simulink model "MX_Gateway"  (Simulink 9.0)
settings: Solver=FixedStepDiscrete  StartTime=0.0  StopTime=100  FixedStep=0.01  RelTol=1e-3
values from neighbouring files (symbols this model uses):
  ACTIVE = Bobcat.Parameter; CreateDefine( ACTIVE                         ,1                          ,'Define' ,'defines.h' )    [defines.m]
  ACTIVE_MODE_ONE = Bobcat.Parameter; CreateDefine( ACTIVE_MODE_ONE                ,19                         ,'Define' ,'defines.h' )    [defines.m]
  AUTHENTICATED = Bobcat.Parameter; CreateDefine( AUTHENTICATED                  ,1                          ,'Define' ,'defines.h' )    [defines.m]
  AUX_EMEA_CONTROLS = Bobcat.Parameter; CreateDefine( AUX_EMEA_CONTROLS  ,1   ,'Define' ,'defines.h' )    [enums.m]
  AUX_FLOW_HIGH = Bobcat.Parameter; CreateDefine( AUX_FLOW_HIGH           ,4  ,'Define' ,'defines.h' )    [enums.m]
  AUX_FLOW_INACTIVE = Bobcat.Parameter; CreateDefine( AUX_FLOW_INACTIVE       ,0  ,'Define' ,'defines.h' )    [enums.m]
  AUX_FLOW_LOW = Bobcat.Parameter; CreateDefine( AUX_FLOW_LOW            ,1  ,'Define' ,'defines.h' )    [enums.m]
  AUX_FLOW_MEDIUM = Bobcat.Parameter; CreateDefine( AUX_FLOW_MEDIUM         ,2  ,'Define' ,'defines.h' )    [enums.m]
  AUX_FLOW_STANDARD = Bobcat.Parameter; CreateDefine( AUX_FLOW_STANDARD       ,3  ,'Define' ,'defines.h' )    [enums.m]
  AUX_NON_FUNCTIONAL = Bobcat.Parameter; CreateDefine( AUX_NON_FUNCTIONAL ,255 ,'Define' ,'defines.h' )    [enums.m]
  BASE_ACTIVE = Bobcat.Parameter; CreateDefine( BASE_ACTIVE                    ,253                        ,'Define' ,'defines.h' )    [defines.m]
  BASE_DETENT = Bobcat.Parameter; CreateDefine( BASE_DETENT                    ,1                          ,'Define' ,'defines.h' )    [defines.m]
  BEEP_NONE = Bobcat.Parameter; CreateDefine( BEEP_NONE                      ,255                        ,'Define' ,'defines.h' )    [defines.m]
  CD_AIR_FILTER_DEBOUNCE_NORMAL = Bobcat.Parameter; CreateAliasCD( CD_AIR_FILTER_DEBOUNCE_NORMAL                        ,uint16(500)                ,'ConstantDataPointer' ,'CONSTANT_DATA' ,'AIR_FILTER_DEBOUNCE_NORMAL')    [cdata.m]    (also in hc12_cdata.m; m4_cdata.m)
  CD_AIR_FILTER_DEBOUNCE_NOT_CONNECTED = Bobcat.Parameter; CreateAliasCD( CD_AIR_FILTER_DEBOUNCE_NOT_CONNECTED                 ,uint16(3000)               ,'ConstantDataPointer' ,'CONSTANT_DATA' ,'AIR_FILTER_DEBOUNCE_NOT_CONNECTED')    [cdata.m]    (also in hc12_cdata.m; m4_cdata.m)
  CD_AIR_FILTER_DEBOUNCE_PLUGGED = Bobcat.Parameter; CreateAliasCD( CD_AIR_FILTER_DEBOUNCE_PLUGGED                       ,uint16(3000)               ,'ConstantDataPointer' ,'CONSTANT_DATA' ,'AIR_FILTER_DEBOUNCE_PLUGGED')    [cdata.m]    (also in hc12_cdata.m; m4_cdata.m)
  CD_AIR_FILTER_FUNCTION = Bobcat.Parameter; CreateAliasCD( CD_AIR_FILTER_FUNCTION                               ,uint8(255)                 ,'ConstantDataPointer' ,'CONSTANT_DATA' ,'AIR_FILTER_FUNCTION')    [cdata.m]    (also in hc12_cdata.m; m4_cdata.m)
  CD_AIR_FILTER_PLUGGED_WEIGHT = Bobcat.Parameter; CreateAliasCD( CD_AIR_FILTER_PLUGGED_WEIGHT                         ,uint8(2)                   ,'ConstantDataPointer' ,'CONSTANT_DATA' ,'AIR_FILTER_PLUGGED_WEIGHT')    [cdata.m]    (also in hc12_cdata.m; m4_cdata.m)
  CD_AIR_FILTER_UNPLUGGED_WEIGHT = Bobcat.Parameter; CreateAliasCD( CD_AIR_FILTER_UNPLUGGED_WEIGHT                       ,uint8(1)                   ,'ConstantDataPointer' ,'CONSTANT_DATA' ,'AIR_FILTER_UNPLUGGED_WEIGHT')    [cdata.m]    (also in hc12_cdata.m; m4_cdata.m)
  CD_AUTOIDLE_ARMBAR_DELAY = Bobcat.Parameter; CreateAliasCD( CD_AUTOIDLE_ARMBAR_DELAY                             ,uint8(2)                   ,'ConstantDataPointer' ,'CONSTANT_DATA' ,'AUTOIDLE_ARMBAR_DELAY')    [cdata.m]    (also in hc12_cdata.m; m4_cdata.m)
  CD_AUTOIDLE_FUNCTION = Bobcat.Parameter; CreateAliasCD( CD_AUTOIDLE_FUNCTION                                 ,uint8(255)                 ,'ConstantDataPointer' ,'CONSTANT_DATA' ,'AUTOIDLE_FUNCTION')    [cdata.m]    (also in hc12_cdata.m; m4_cdata.m)
  CD_AUTOIDLE_IDLE_DELAY = Bobcat.Parameter; CreateAliasCD( CD_AUTOIDLE_IDLE_DELAY                               ,uint8(40)                  ,'ConstantDataPointer' ,'CONSTANT_DATA' ,'AUTOIDLE_IDLE_DELAY')    [cdata.m]    (also in hc12_cdata.m; m4_cdata.m)
  CD_AUTOIDLE_LOW_IDLE_START = Bobcat.Parameter; CreateAliasCD( CD_AUTOIDLE_LOW_IDLE_START                           ,uint8(255)                 ,'ConstantDataPointer' ,'CONSTANT_DATA' ,'AUTOIDLE_LOW_IDLE_START')    [cdata.m]    (also in hc12_cdata.m; m4_cdata.m)
  CD_AUTOIDLE_PRESSURE_OFF = Bobcat.Parameter; CreateAliasCD( CD_AUTOIDLE_PRESSURE_OFF                             ,uint16(300)                ,'ConstantDataPointer' ,'CONSTANT_DATA' ,'AUTOIDLE_PRESSURE_OFF')    [cdata.m]    (also in hc12_cdata.m; m4_cdata.m)
  CD_AUTOIDLE_PRESSURE_ON = Bobcat.Parameter; CreateAliasCD( CD_AUTOIDLE_PRESSURE_ON                              ,uint16(270)                ,'ConstantDataPointer' ,'CONSTANT_DATA' ,'AUTOIDLE_PRESSURE_ON')    [cdata.m]    (also in hc12_cdata.m; m4_cdata.m)
  CD_AUTOSHIFT_FUNCTION = Bobcat.Parameter; CreateAliasCD( CD_AUTOSHIFT_FUNCTION                                ,uint8(255)                 ,'ConstantDataPointer' ,'CONSTANT_DATA' ,'AUTOSHIFT_FUNCTION')    [cdata.m]    (also in hc12_cdata.m; m4_cdata.m)
  CD_AUTOSHIFT_PHIGH1 = Bobcat.Parameter; CreateAliasCD( CD_AUTOSHIFT_PHIGH1                                  ,uint16(205)                ,'ConstantDataPointer' ,'CONSTANT_DATA' ,'AUTOSHIFT_PHIGH1')    [cdata.m]    (also in hc12_cdata.m; m4_cdata.m)
  CD_AUTOSHIFT_PHIGH2 = Bobcat.Parameter; CreateAliasCD( CD_AUTOSHIFT_PHIGH2                                  ,uint16(193)                ,'ConstantDataPointer' ,'CONSTANT_DATA' ,'AUTOSHIFT_PHIGH2')    [cdata.m]    (also in hc12_cdata.m; m4_cdata.m)
  CD_AUTOSHIFT_PHIGH_TEMP1 = Bobcat.Parameter; CreateAliasCD( CD_AUTOSHIFT_PHIGH_TEMP1                             ,int16(70)                  ,'ConstantDataPointer' ,'CONSTANT_DATA' ,'AUTOSHIFT_PHIGH_TEMP1')    [cdata.m]    (also in hc12_cdata.m; m4_cdata.m)
  CD_AUTOSHIFT_PHIGH_TEMP2 = Bobcat.Parameter; CreateAliasCD( CD_AUTOSHIFT_PHIGH_TEMP2                             ,int16(160)                 ,'ConstantDataPointer' ,'CONSTANT_DATA' ,'AUTOSHIFT_PHIGH_TEMP2')    [cdata.m]    (also in hc12_cdata.m; m4_cdata.m)
  CD_AUTOSHIFT_PLOW1 = Bobcat.Parameter; CreateAliasCD( CD_AUTOSHIFT_PLOW1                                   ,uint16(130)                ,'ConstantDataPointer' ,'CONSTANT_DATA' ,'AUTOSHIFT_PLOW1')    [cdata.m]    (also in hc12_cdata.m; m4_cdata.m)
  CD_AUTOSHIFT_PLOW2 = Bobcat.Parameter; CreateAliasCD( CD_AUTOSHIFT_PLOW2                                   ,uint16(100)                ,'ConstantDataPointer' ,'CONSTANT_DATA' ,'AUTOSHIFT_PLOW2')    [cdata.m]    (also in hc12_cdata.m; m4_cdata.m)
  CD_AUTOSHIFT_PLOW_TEMP1 = Bobcat.Parameter; CreateAliasCD( CD_AUTOSHIFT_PLOW_TEMP1                              ,int16(20)                  ,'ConstantDataPointer' ,'CONSTANT_DATA' ,'AUTOSHIFT_PLOW_TEMP1')    [cdata.m]    (also in hc12_cdata.m; m4_cdata.m)
  CD_AUTOSHIFT_PLOW_TEMP2 = Bobcat.Parameter; CreateAliasCD( CD_AUTOSHIFT_PLOW_TEMP2                              ,int16(80)                  ,'ConstantDataPointer' ,'CONSTANT_DATA' ,'AUTOSHIFT_PLOW_TEMP2')    [cdata.m]    (also in hc12_cdata.m; m4_cdata.m)
  CD_AUTOSHIFT_TRAVEL_DELAY = Bobcat.Parameter; CreateAliasCD( CD_AUTOSHIFT_TRAVEL_DELAY                            ,uint8(32)                  ,'ConstantDataPointer' ,'CONSTANT_DATA' ,'AUTOSHIFT_TRAVEL_DELAY')    [cdata.m]    (also in hc12_cdata.m; m4_cdata.m)
  CD_AUX2_CRACKPOINT_CURRENT = Bobcat.Parameter; CreateAliasCD( CD_AUX2_CRACKPOINT_CURRENT                           ,fi(0,0,16,12,'hex','0c28') ,'ConstantDataPointer' ,'CONSTANT_DATA' ,'AUX2_CRACKPOINT_CURRENT')    [cdata.m]    (also in hc12_cdata.m; m4_cdata.m)
  CD_AUX2_FLOW_LOW_FUNCTION = Bobcat.Parameter; CreateAliasCD( CD_AUX2_FLOW_LOW_FUNCTION                            ,uint8(255)                 ,'ConstantDataPointer' ,'CONSTANT_DATA' ,'AUX2_FLOW_LOW_FUNCTION')    [cdata.m]    (also in hc12_cdata.m; m4_cdata.m)
  CD_AUX2_FLOW_MED_FUNCTION = Bobcat.Parameter; CreateAliasCD( CD_AUX2_FLOW_MED_FUNCTION                            ,uint8(255)                 ,'ConstantDataPointer' ,'CONSTANT_DATA' ,'AUX2_FLOW_MED_FUNCTION')    [cdata.m]    (also in hc12_cdata.m; m4_cdata.m)
  CD_AUX2_HI_FLOW_PERCENT = Bobcat.Parameter; CreateAliasCD( CD_AUX2_HI_FLOW_PERCENT                              ,fi(0,0,16,16,'hex','ffff') ,'ConstantDataPointer' ,'CONSTANT_DATA' ,'AUX2_HI_FLOW_PERCENT')    [cdata.m]    (also in hc12_cdata.m; m4_cdata.m)
  CD_AUX2_LOW_FLOW_PERCENT = Bobcat.Parameter; CreateAliasCD( CD_AUX2_LOW_FLOW_PERCENT                             ,fi(0,0,16,16,'hex','7333') ,'ConstantDataPointer' ,'CONSTANT_DATA' ,'AUX2_LOW_FLOW_PERCENT')    [cdata.m]    (also in hc12_cdata.m; m4_cdata.m)
  CD_AUX2_MAX_FLOW_CURRENT = Bobcat.Parameter; CreateAliasCD( CD_AUX2_MAX_FLOW_CURRENT                             ,fi(0,0,16,12,'hex','14cc') ,'ConstantDataPointer' ,'CONSTANT_DATA' ,'AUX2_MAX_FLOW_CURRENT')    [cdata.m]    (also in hc12_cdata.m; m4_cdata.m)
  CD_AUX2_MED_FLOW_PERCENT = Bobcat.Parameter; CreateAliasCD( CD_AUX2_MED_FLOW_PERCENT                             ,fi(0,0,16,16,'hex','9999') ,'ConstantDataPointer' ,'CONSTANT_DATA' ,'AUX2_MED_FLOW_PERCENT')    [cdata.m]    (also in hc12_cdata.m; m4_cdata.m)
  CD_AUX_CRACKPOINT_CURRENT = Bobcat.Parameter; CreateAliasCD( CD_AUX_CRACKPOINT_CURRENT                            ,fi(0,0,16,12,'hex','0000') ,'ConstantDataPointer' ,'CONSTANT_DATA' ,'AUX_CRACKPOINT_CURRENT')    [cdata.m]    (also in hc12_cdata.m; m4_cdata.m)
  CD_AUX_DETENT_FLASH_TIME = Bobcat.Parameter; CreateAliasCD( CD_AUX_DETENT_FLASH_TIME                             ,uint16(72)                 ,'ConstantDataPointer' ,'CONSTANT_DATA' ,'AUX_DETENT_FLASH_TIME')    [cdata.m]    (also in hc12_cdata.m; m4_cdata.m)
  CD_AUX_DETENT_HOLD_TIME = Bobcat.Parameter; CreateAliasCD( CD_AUX_DETENT_HOLD_TIME                              ,uint16(100)                ,'ConstantDataPointer' ,'CONSTANT_DATA' ,'AUX_DETENT_HOLD_TIME')    [cdata.m]    (also in hc12_cdata.m; m4_cdata.m)
  CD_AUX_FULL_ON_AUX1 = Bobcat.Parameter; CreateAliasCD( CD_AUX_FULL_ON_AUX1                                  ,fi(0,0,16,12,'hex','10cd') ,'ConstantDataPointer' ,'CONSTANT_DATA' ,'AUX_FULL_ON_AUX1')    [cdata.m]    (also in hc12_cdata.m; m4_cdata.m)
  CD_AUX_FULL_ON_AUX2 = Bobcat.Parameter; CreateAliasCD( CD_AUX_FULL_ON_AUX2                                  ,fi(0,0,16,12,'hex','139d') ,'ConstantDataPointer' ,'CONSTANT_DATA' ,'AUX_FULL_ON_AUX2')    [cdata.m]    (also in hc12_cdata.m; m4_cdata.m)
  CD_AUX_FULL_ON_AUX3 = Bobcat.Parameter; CreateAliasCD( CD_AUX_FULL_ON_AUX3                                  ,fi(0,0,16,12,'hex','17d7') ,'ConstantDataPointer' ,'CONSTANT_DATA' ,'AUX_FULL_ON_AUX3')    [cdata.m]    (also in hc12_cdata.m; m4_cdata.m)
  CD_AUX_FUNCTION = Bobcat.Parameter; CreateAliasCD( CD_AUX_FUNCTION                                      ,uint8(0)                   ,'ConstantDataPointer' ,'CONSTANT_DATA' ,'AUX_FUNCTION')    [cdata.m]    (also in hc12_cdata.m; m4_cdata.m)
  CD_AUX_RATE_LIMIT_CRACKPOINT = Bobcat.Parameter; CreateAliasCD( CD_AUX_RATE_LIMIT_CRACKPOINT                         ,fi(0,0,16,12,'hex','0800') ,'ConstantDataPointer' ,'CONSTANT_DATA' ,'AUX_RATE_LIMIT_CRACKPOINT')    [cdata.m]    (also in hc12_cdata.m; m4_cdata.m)
  CD_AUX_RATE_LIMIT_CRACKPOINT_RATE = Bobcat.Parameter; CreateAliasCD( CD_AUX_RATE_LIMIT_CRACKPOINT_RATE                    ,fi(0,1,32,12,'int',102400) ,'ConstantDataPointer' ,'CONSTANT_DATA' ,'AUX_RATE_LIMIT_CRACKPOINT_RATE')    [cdata.m]    (also in hc12_cdata.m; m4_cdata.m)
  CD_AUX_RATE_LIMIT_FUNCTION = Bobcat.Parameter; CreateAliasCD( CD_AUX_RATE_LIMIT_FUNCTION                           ,uint8(255)                 ,'ConstantDataPointer' ,'CONSTANT_DATA' ,'AUX_RATE_LIMIT_FUNCTION')    [cdata.m]    (also in hc12_cdata.m; m4_cdata.m)
  CD_AUX_RATE_LIMIT_MAX_ENG_SPD = Bobcat.Parameter; CreateAliasCD( CD_AUX_RATE_LIMIT_MAX_ENG_SPD                        ,uint16(1600)               ,'ConstantDataPointer' ,'CONSTANT_DATA' ,'AUX_RATE_LIMIT_MAX_ENG_SPD')    [cdata.m]    (also in hc12_cdata.m; m4_cdata.m)
  CD_AUX_RATE_LIMIT_MIN_ENG_SPD = Bobcat.Parameter; CreateAliasCD( CD_AUX_RATE_LIMIT_MIN_ENG_SPD                        ,uint16(100)                ,'ConstantDataPointer' ,'CONSTANT_DATA' ,'AUX_RATE_LIMIT_MIN_ENG_SPD')    [cdata.m]    (also in hc12_cdata.m; m4_cdata.m)
  CD_AUX_RATE_LIMIT_NORMAL_RATE = Bobcat.Parameter; CreateAliasCD( CD_AUX_RATE_LIMIT_NORMAL_RATE                        ,fi(0,1,32,12,'int',2048)   ,'ConstantDataPointer' ,'CONSTANT_DATA' ,'AUX_RATE_LIMIT_NORMAL_RATE')    [cdata.m]    (also in hc12_cdata.m; m4_cdata.m)
  CD_AUXPOT_CAL_DEADBAND_HIGH = Bobcat.Parameter; CreateAliasCD( CD_AUXPOT_CAL_DEADBAND_HIGH                          ,uint16(574)                ,'ConstantDataPointer' ,'CONSTANT_DATA' ,'AUXPOT_CAL_DEADBAND_HIGH')    [cdata.m]    (also in hc12_cdata.m; m4_cdata.m)
  CD_AUXPOT_CAL_DEADBAND_LOW = Bobcat.Parameter; CreateAliasCD( CD_AUXPOT_CAL_DEADBAND_LOW                           ,uint16(424)                ,'ConstantDataPointer' ,'CONSTANT_DATA' ,'AUXPOT_CAL_DEADBAND_LOW')    [cdata.m]    (also in hc12_cdata.m; m4_cdata.m)
  CD_AUXPOT_CENTER_RATIO = Bobcat.Parameter; CreateAliasCD( CD_AUXPOT_CENTER_RATIO                               ,uint16(500)                ,'ConstantDataPointer' ,'CONSTANT_DATA' ,'AUXPOT_CENTER_RATIO')    [cdata.m]    (also in hc12_cdata.m; m4_cdata.m)
  CD_AUXPOT_DEADBAND = Bobcat.Parameter; CreateAliasCD( CD_AUXPOT_DEADBAND                                   ,uint8(38)                  ,'ConstantDataPointer' ,'CONSTANT_DATA' ,'AUXPOT_DEADBAND')    [cdata.m]    (also in hc12_cdata.m; m4_cdata.m)
  CD_AUXPOT_FUNCTION = Bobcat.Parameter; CreateAliasCD( CD_AUXPOT_FUNCTION                                   ,uint8(0)                   ,'ConstantDataPointer' ,'CONSTANT_DATA' ,'AUXPOT_FUNCTION')    [cdata.m]    (also in hc12_cdata.m; m4_cdata.m)
  CD_AUXPOT_RATIO_GAIN = Bobcat.Parameter; CreateAliasCD( CD_AUXPOT_RATIO_GAIN                                 ,uint16(1000)               ,'ConstantDataPointer' ,'CONSTANT_DATA' ,'AUXPOT_RATIO_GAIN')    [cdata.m]    (also in hc12_cdata.m; m4_cdata.m)
  CD_CAN_THROTTLE_FUNCTION = Bobcat.Parameter; CreateAliasCD( CD_CAN_THROTTLE_FUNCTION                             ,uint8(255)                 ,'ConstantDataPointer' ,'CONSTANT_DATA' ,'CAN_THROTTLE_FUNCTION')    [cdata.m]    (also in hc12_cdata.m; m4_cdata.m)
  CD_CAN_THROTTLE_REVERSE = Bobcat.Parameter; CreateAliasCD( CD_CAN_THROTTLE_REVERSE                              ,uint8(255)                 ,'ConstantDataPointer' ,'CONSTANT_DATA' ,'CAN_THROTTLE_REVERSE')    [cdata.m]    (also in hc12_cdata.m; m4_cdata.m)
  CD_CONSOLE_DEBOUNCE_ABSENT = Bobcat.Parameter; CreateAliasCD( CD_CONSOLE_DEBOUNCE_ABSENT                           ,uint16(12)                 ,'ConstantDataPointer' ,'CONSTANT_DATA' ,'CONSOLE_DEBOUNCE_ABSENT')    [cdata.m]    (also in hc12_cdata.m; m4_cdata.m)
  CD_CONSOLE_DEBOUNCE_OORH = Bobcat.Parameter; CreateAliasCD( CD_CONSOLE_DEBOUNCE_OORH                             ,uint16(12)                 ,'ConstantDataPointer' ,'CONSTANT_DATA' ,'CONSOLE_DEBOUNCE_OORH')    [cdata.m]    (also in hc12_cdata.m; m4_cdata.m)
  CD_CONSOLE_DEBOUNCE_OORL = Bobcat.Parameter; CreateAliasCD( CD_CONSOLE_DEBOUNCE_OORL                             ,uint16(12)                 ,'ConstantDataPointer' ,'CONSTANT_DATA' ,'CONSOLE_DEBOUNCE_OORL')    [cdata.m]    (also in hc12_cdata.m; m4_cdata.m)
  CD_CONSOLE_DEBOUNCE_PRESENT = Bobcat.Parameter; CreateAliasCD( CD_CONSOLE_DEBOUNCE_PRESENT                          ,uint16(12)                 ,'ConstantDataPointer' ,'CONSTANT_DATA' ,'CONSOLE_DEBOUNCE_PRESENT')    [cdata.m]    (also in hc12_cdata.m; m4_cdata.m)
  CD_CONSOLE_FUNCTION = Bobcat.Parameter; CreateAliasCD( CD_CONSOLE_FUNCTION                                  ,uint8(0)                   ,'ConstantDataPointer' ,'CONSTANT_DATA' ,'CONSOLE_FUNCTION')    [cdata.m]    (also in hc12_cdata.m; m4_cdata.m)
  CD_CONSOLE_OORH = Bobcat.Parameter; CreateAliasCD( CD_CONSOLE_OORH                                      ,uint16(740)                ,'ConstantDataPointer' ,'CONSTANT_DATA' ,'CONSOLE_OORH')    [cdata.m]    (also in hc12_cdata.m; m4_cdata.m)
  CD_CONSOLE_OORL = Bobcat.Parameter; CreateAliasCD( CD_CONSOLE_OORL                                      ,uint16(10)                 ,'ConstantDataPointer' ,'CONSTANT_DATA' ,'CONSOLE_OORL')    [cdata.m]    (also in hc12_cdata.m; m4_cdata.m)
  CD_CONSOLE_PRESENT_HIGH = Bobcat.Parameter; CreateAliasCD( CD_CONSOLE_PRESENT_HIGH                              ,uint16(515)                ,'ConstantDataPointer' ,'CONSTANT_DATA' ,'CONSOLE_PRESENT_HIGH')    [cdata.m]    (also in hc12_cdata.m; m4_cdata.m)
  CD_CONSOLE_PRESENT_LOW = Bobcat.Parameter; CreateAliasCD( CD_CONSOLE_PRESENT_LOW                               ,uint16(375)                ,'ConstantDataPointer' ,'CONSTANT_DATA' ,'CONSOLE_PRESENT_LOW')    [cdata.m]    (also in hc12_cdata.m; m4_cdata.m)
  CD_DELUXE_GEN5_5_FUNCTION = Bobcat.Parameter; CreateAliasCD( CD_DELUXE_GEN5_5_FUNCTION                            ,uint8(255)                 ,'ConstantDataPointer' ,'CONSTANT_DATA' ,'DELUXE_GEN5_5_FUNCTION')    [cdata.m]    (also in hc12_cdata.m; m4_cdata.m)
  CD_DELUXE_GEN5_FUNCTION = Bobcat.Parameter; CreateAliasCD( CD_DELUXE_GEN5_FUNCTION                              ,uint8(255)                 ,'ConstantDataPointer' ,'CONSTANT_DATA' ,'DELUXE_GEN5_FUNCTION')    [cdata.m]    (also in hc12_cdata.m; m4_cdata.m)
  CD_DERATE_ENGINE_SPEED_DEBOUNCE_HIGH = Bobcat.Parameter; CreateAliasCD( CD_DERATE_ENGINE_SPEED_DEBOUNCE_HIGH                 ,uint16(200)                ,'ConstantDataPointer' ,'CONSTANT_DATA' ,'DERATE_ENGINE_SPEED_DEBOUNCE_HIGH')    [cdata.m]    (also in hc12_cdata.m; m4_cdata.m)
  CD_DERATE_ENGINE_SPEED_DEBOUNCE_SHUTDOWN = Bobcat.Parameter; CreateAliasCD( CD_DERATE_ENGINE_SPEED_DEBOUNCE_SHUTDOWN             ,uint16(1000)               ,'ConstantDataPointer' ,'CONSTANT_DATA' ,'DERATE_ENGINE_SPEED_DEBOUNCE_SHUTDOWN')    [cdata.m]    (also in hc12_cdata.m; m4_cdata.m)
  CD_DERATE_ENGINE_SPEED_HIGH_OFFSET = Bobcat.Parameter; CreateAliasCD( CD_DERATE_ENGINE_SPEED_HIGH_OFFSET                   ,uint16(200)                ,'ConstantDataPointer' ,'CONSTANT_DATA' ,'DERATE_ENGINE_SPEED_HIGH_OFFSET')    [cdata.m]    (also in hc12_cdata.m; m4_cdata.m)
  CD_DERATE_METHOD = Bobcat.Parameter; CreateAliasCD( CD_DERATE_METHOD                                     ,uint8(0)                   ,'ConstantDataPointer' ,'CONSTANT_DATA' ,'DERATE_METHOD')    [cdata.m]    (also in hc12_cdata.m; m4_cdata.m)
  CD_DUMP_VALVE_FUNCTION = Bobcat.Parameter; CreateAliasCD( CD_DUMP_VALVE_FUNCTION                               ,uint8(255)                 ,'ConstantDataPointer' ,'CONSTANT_DATA' ,'DUMP_VALVE_FUNCTION')    [cdata.m]    (also in hc12_cdata.m; m4_cdata.m)
  CD_DUMP_VALVE_ON_CURRENT = Bobcat.Parameter; CreateAliasCD( CD_DUMP_VALVE_ON_CURRENT                             ,fi(0,0,16,12,'hex','ffff') ,'ConstantDataPointer' ,'CONSTANT_DATA' ,'DUMP_VALVE_ON_CURRENT')    [cdata.m]    (also in hc12_cdata.m; m4_cdata.m)
  CD_ECO_MODE_THROTTLE_REDUCTION = Bobcat.Parameter; CreateAliasCD( CD_ECO_MODE_THROTTLE_REDUCTION                       ,uint16(850)                ,'ConstantDataPointer' ,'CONSTANT_DATA' ,'ECO_MODE_THROTTLE_REDUCTION')    [cdata.m]    (also in hc12_cdata.m; m4_cdata.m)
  CD_ECT_FILTER_GAIN = Bobcat.Parameter; CreateAliasCD( CD_ECT_FILTER_GAIN                                   ,uint8(6)                   ,'ConstantDataPointer' ,'CONSTANT_DATA' ,'ECT_FILTER_GAIN')    [cdata.m]    (also in hc12_cdata.m; m4_cdata.m)
  CD_ECU_FUNCTION = Bobcat.Parameter; CreateAliasCD( CD_ECU_FUNCTION                                      ,uint8(255)                 ,'ConstantDataPointer' ,'CONSTANT_DATA' ,'ECU_FUNCTION')    [cdata.m]    (also in hc12_cdata.m; m4_cdata.m)
  CD_ECU_HOLD_DEBOUNCE_ERROR_ON = Bobcat.Parameter; CreateAliasCD( CD_ECU_HOLD_DEBOUNCE_ERROR_ON                        ,uint16(20)                 ,'ConstantDataPointer' ,'CONSTANT_DATA' ,'ECU_HOLD_DEBOUNCE_ERROR_ON')    [cdata.m]    (also in hc12_cdata.m; m4_cdata.m)
  CD_EMEA_CONTROLS_HOLD_TIME = Bobcat.Parameter; CreateAliasCD( CD_EMEA_CONTROLS_HOLD_TIME                           ,uint16(30)                 ,'ConstantDataPointer' ,'CONSTANT_DATA' ,'EMEA_CONTROLS_HOLD_TIME')    [cdata.m]    (also in hc12_cdata.m; m4_cdata.m)
  CD_EMEA_CONTROLS_OPTION_A = Bobcat.Parameter; CreateAliasCD( CD_EMEA_CONTROLS_OPTION_A                            ,uint8(255)                 ,'ConstantDataPointer' ,'CONSTANT_DATA' ,'EMEA_CONTROLS_OPTION_A')    [cdata.m]    (also in hc12_cdata.m; m4_cdata.m)
  CD_EMEA_CONTROLS_OPTION_C = Bobcat.Parameter; CreateAliasCD( CD_EMEA_CONTROLS_OPTION_C                            ,uint8(255)                 ,'ConstantDataPointer' ,'CONSTANT_DATA' ,'EMEA_CONTROLS_OPTION_C')    [cdata.m]    (also in hc12_cdata.m; m4_cdata.m)
  CD_EMEA_CONTROLS_REVERSE = Bobcat.Parameter; CreateAliasCD( CD_EMEA_CONTROLS_REVERSE                             ,uint8(255)                 ,'ConstantDataPointer' ,'CONSTANT_DATA' ,'EMEA_CONTROLS_REVERSE')    [cdata.m]    (also in hc12_cdata.m; m4_cdata.m)
  CD_EMEA_ELECTRIC_VERSION = Bobcat.Parameter; CreateAliasCD( CD_EMEA_ELECTRIC_VERSION                             ,uint8(0)                   ,'ConstantDataPointer' ,'CONSTANT_DATA' ,'EMEA_ELECTRIC_VERSION')    [cdata.m]    (also in hc12_cdata.m; m4_cdata.m)
  CD_EMEA_POT_CAL_DEADBAND_HIGH = Bobcat.Parameter; CreateAliasCD( CD_EMEA_POT_CAL_DEADBAND_HIGH                        ,uint16(574)                ,'ConstantDataPointer' ,'CONSTANT_DATA' ,'EMEA_POT_CAL_DEADBAND_HIGH')    [cdata.m]    (also in hc12_cdata.m; m4_cdata.m)
  CD_EMEA_POT_CAL_DEADBAND_LOW = Bobcat.Parameter; CreateAliasCD( CD_EMEA_POT_CAL_DEADBAND_LOW                         ,uint16(424)                ,'ConstantDataPointer' ,'CONSTANT_DATA' ,'EMEA_POT_CAL_DEADBAND_LOW')    [cdata.m]    (also in hc12_cdata.m; m4_cdata.m)
  CD_ENGINE_RPM_FACTOR = Bobcat.Parameter; CreateAliasCD( CD_ENGINE_RPM_FACTOR                                 ,uint8(35)                  ,'ConstantDataPointer' ,'CONSTANT_DATA' ,'ENGINE_RPM_FACTOR')    [cdata.m]    (also in hc12_cdata.m; m4_cdata.m)
  CD_ENGINE_SPEED_FILTER_FAST_VALUE = Bobcat.Parameter; CreateAliasCD( CD_ENGINE_SPEED_FILTER_FAST_VALUE                    ,uint8(1)                   ,'ConstantDataPointer' ,'CONSTANT_DATA' ,'ENGINE_SPEED_FILTER_FAST_VALUE')    [cdata.m]    (also in hc12_cdata.m; m4_cdata.m)
  CD_ENGINE_SPEED_FILTER_SLOW_VALUE = Bobcat.Parameter; CreateAliasCD( CD_ENGINE_SPEED_FILTER_SLOW_VALUE                    ,uint8(4)                   ,'ConstantDataPointer' ,'CONSTANT_DATA' ,'ENGINE_SPEED_FILTER_SLOW_VALUE')    [cdata.m]    (also in hc12_cdata.m; m4_cdata.m)
  CD_ENGINE_SPEED_RUNNING = Bobcat.Parameter; CreateAliasCD( CD_ENGINE_SPEED_RUNNING                              ,uint16(800)                ,'ConstantDataPointer' ,'CONSTANT_DATA' ,'ENGINE_SPEED_RUNNING')    [cdata.m]    (also in hc12_cdata.m; m4_cdata.m)
  CD_ENGINE_START_DELAY_DEBOUNCE_RPM_NO_SIGNAL = Bobcat.Parameter; CreateAliasCD( CD_ENGINE_START_DELAY_DEBOUNCE_RPM_NO_SIGNAL         ,uint16(0)                  ,'ConstantDataPointer' ,'CONSTANT_DATA' ,'ENGINE_START_DELAY_DEBOUNCE_RPM_NO_SIGNAL')    [cdata.m]    (also in hc12_cdata.m; m4_cdata.m)
  CD_ENGINE_START_DELAY_OFF_TIME = Bobcat.Parameter; CreateAliasCD( CD_ENGINE_START_DELAY_OFF_TIME                       ,uint16(0)                  ,'ConstantDataPointer' ,'CONSTANT_DATA' ,'ENGINE_START_DELAY_OFF_TIME')    [cdata.m]    (also in hc12_cdata.m; m4_cdata.m)
  CD_ENGINE_START_DELAY_RPM_NO_SIGNAL = Bobcat.Parameter; CreateAliasCD( CD_ENGINE_START_DELAY_RPM_NO_SIGNAL                  ,uint16(0)                  ,'ConstantDataPointer' ,'CONSTANT_DATA' ,'ENGINE_START_DELAY_RPM_NO_SIGNAL')    [cdata.m]    (also in hc12_cdata.m; m4_cdata.m)
  CD_EOP_DEBOUNCE_EXTREMELY_LOW = Bobcat.Parameter; CreateAliasCD( CD_EOP_DEBOUNCE_EXTREMELY_LOW                        ,uint16(50)                 ,'ConstantDataPointer' ,'CONSTANT_DATA' ,'EOP_DEBOUNCE_EXTREMELY_LOW')    [cdata.m]    (also in hc12_cdata.m; m4_cdata.m)
  CD_EOP_DEBOUNCE_NORMAL = Bobcat.Parameter; CreateAliasCD( CD_EOP_DEBOUNCE_NORMAL                               ,uint16(50)                 ,'ConstantDataPointer' ,'CONSTANT_DATA' ,'EOP_DEBOUNCE_NORMAL')    [cdata.m]    (also in hc12_cdata.m; m4_cdata.m)
  CD_EOP_DEBOUNCE_SHUTDOWN = Bobcat.Parameter; CreateAliasCD( CD_EOP_DEBOUNCE_SHUTDOWN                             ,uint16(100)                ,'ConstantDataPointer' ,'CONSTANT_DATA' ,'EOP_DEBOUNCE_SHUTDOWN')    [cdata.m]    (also in hc12_cdata.m; m4_cdata.m)
  CD_EOP_FILTER_VALUE = Bobcat.Parameter; CreateAliasCD( CD_EOP_FILTER_VALUE                                  ,uint8(3)                   ,'ConstantDataPointer' ,'CONSTANT_DATA' ,'EOP_FILTER_VALUE')    [cdata.m]    (also in hc12_cdata.m; m4_cdata.m)
  CD_EOP_FUNCTION = Bobcat.Parameter; CreateAliasCD( CD_EOP_FUNCTION                                      ,uint8(1)                   ,'ConstantDataPointer' ,'CONSTANT_DATA' ,'EOP_FUNCTION')    [cdata.m]    (also in hc12_cdata.m; m4_cdata.m)
  CD_EOP_SWITCH_GAUGE_VALUE = Bobcat.Parameter; CreateAliasCD( CD_EOP_SWITCH_GAUGE_VALUE                            ,uint16(125)                ,'ConstantDataPointer' ,'CONSTANT_DATA' ,'EOP_SWITCH_GAUGE_VALUE')    [cdata.m]    (also in hc12_cdata.m; m4_cdata.m)
  CD_EOP_SWITCH_LOW = Bobcat.Parameter; CreateAliasCD( CD_EOP_SWITCH_LOW                                    ,uint16(50)                 ,'ConstantDataPointer' ,'CONSTANT_DATA' ,'EOP_SWITCH_LOW')    [cdata.m]    (also in hc12_cdata.m; m4_cdata.m)
  CD_EOP_SWITCH_PRESSURE_VALUE = Bobcat.Parameter; CreateAliasCD( CD_EOP_SWITCH_PRESSURE_VALUE                         ,uint16(40)                 ,'ConstantDataPointer' ,'CONSTANT_DATA' ,'EOP_SWITCH_PRESSURE_VALUE')    [cdata.m]    (also in hc12_cdata.m; m4_cdata.m)
  CD_EOT_DEBOUNCE_EXTREMELY_LOW = Bobcat.Parameter; CreateAliasCD( CD_EOT_DEBOUNCE_EXTREMELY_LOW                        ,uint16(2)                  ,'ConstantDataPointer' ,'CONSTANT_DATA' ,'EOT_DEBOUNCE_EXTREMELY_LOW')    [cdata.m]    (also in hc12_cdata.m; m4_cdata.m)
  CD_EOT_DEBOUNCE_LOW = Bobcat.Parameter; CreateAliasCD( CD_EOT_DEBOUNCE_LOW                                  ,uint16(2)                  ,'ConstantDataPointer' ,'CONSTANT_DATA' ,'EOT_DEBOUNCE_LOW')    [cdata.m]    (also in hc12_cdata.m; m4_cdata.m)
  CD_EOT_DEBOUNCE_NORMAL = Bobcat.Parameter; CreateAliasCD( CD_EOT_DEBOUNCE_NORMAL                               ,uint16(2)                  ,'ConstantDataPointer' ,'CONSTANT_DATA' ,'EOT_DEBOUNCE_NORMAL')    [cdata.m]    (also in hc12_cdata.m; m4_cdata.m)
  CD_EOT_EXTREMELY_LOW = Bobcat.Parameter; CreateAliasCD( CD_EOT_EXTREMELY_LOW                                 ,uint16(81)                 ,'ConstantDataPointer' ,'CONSTANT_DATA' ,'EOT_EXTREMELY_LOW')    [cdata.m]    (also in hc12_cdata.m; m4_cdata.m)
  CD_EOT_FUNCTION = Bobcat.Parameter; CreateAliasCD( CD_EOT_FUNCTION                                      ,uint8(255)                 ,'ConstantDataPointer' ,'CONSTANT_DATA' ,'EOT_FUNCTION')    [cdata.m]    (also in hc12_cdata.m; m4_cdata.m)
  CD_EOT_LOW = Bobcat.Parameter; CreateAliasCD( CD_EOT_LOW                                           ,uint16(90)                 ,'ConstantDataPointer' ,'CONSTANT_DATA' ,'EOT_LOW')    [cdata.m]    (also in hc12_cdata.m; m4_cdata.m)
  CD_EOT_TEMPERATURE_HYSTERESIS = Bobcat.Parameter; CreateAliasCD( CD_EOT_TEMPERATURE_HYSTERESIS                        ,uint16(2)                  ,'ConstantDataPointer' ,'CONSTANT_DATA' ,'EOT_TEMPERATURE_HYSTERESIS')    [cdata.m]    (also in hc12_cdata.m; m4_cdata.m)
  CD_FAST_TRAVEL_FUNCTION = Bobcat.Parameter; CreateAliasCD( CD_FAST_TRAVEL_FUNCTION                              ,uint8(255)                 ,'ConstantDataPointer' ,'CONSTANT_DATA' ,'FAST_TRAVEL_FUNCTION')    [cdata.m]    (also in hc12_cdata.m; m4_cdata.m)
  CD_FAST_TRAVEL_THROTTLE_DELAY = Bobcat.Parameter; CreateAliasCD( CD_FAST_TRAVEL_THROTTLE_DELAY                        ,uint16(10)                 ,'ConstantDataPointer' ,'CONSTANT_DATA' ,'FAST_TRAVEL_THROTTLE_DELAY')    [cdata.m]    (also in hc12_cdata.m; m4_cdata.m)
  CD_FAST_TRAVEL_THROTTLE_LIMIT = Bobcat.Parameter; CreateAliasCD( CD_FAST_TRAVEL_THROTTLE_LIMIT                        ,uint16(1000)               ,'ConstantDataPointer' ,'CONSTANT_DATA' ,'FAST_TRAVEL_THROTTLE_LIMIT')    [cdata.m]    (also in hc12_cdata.m; m4_cdata.m)
  CD_FLYWHEEL_TEETH = Bobcat.Parameter; CreateAliasCD( CD_FLYWHEEL_TEETH                                    ,uint8(83)                  ,'ConstantDataPointer' ,'CONSTANT_DATA' ,'FLYWHEEL_TEETH')    [cdata.m]    (also in hc12_cdata.m; m4_cdata.m)
  CD_FUEL_LEVEL_FILTER_FAST_VALUE = Bobcat.Parameter; CreateAliasCD( CD_FUEL_LEVEL_FILTER_FAST_VALUE                      ,uint8(5)                   ,'ConstantDataPointer' ,'CONSTANT_DATA' ,'FUEL_LEVEL_FILTER_FAST_VALUE')    [cdata.m]    (also in hc12_cdata.m; m4_cdata.m)
  CD_FUEL_LEVEL_FILTER_SLOW_VALUE = Bobcat.Parameter; CreateAliasCD( CD_FUEL_LEVEL_FILTER_SLOW_VALUE                      ,uint8(10)                  ,'ConstantDataPointer' ,'CONSTANT_DATA' ,'FUEL_LEVEL_FILTER_SLOW_VALUE')    [cdata.m]    (also in hc12_cdata.m; m4_cdata.m)
  CD_FUEL_PRIMARY_FEEDBACK_FUNCTION = Bobcat.Parameter; CreateAliasCD( CD_FUEL_PRIMARY_FEEDBACK_FUNCTION                    ,uint8(0)                   ,'ConstantDataPointer' ,'CONSTANT_DATA' ,'FUEL_PRIMARY_FEEDBACK_FUNCTION')    [cdata.m]    (also in hc12_cdata.m; m4_cdata.m)
  ... (+246 more)
undefined symbols (not defined in the file or in its folder): sigbuilder_block
undefined symbols (not defined in the file): CD_FUEL_RESOLUTION_TIMER
libraries: simulink x186, MX_Library x107
(part 20 of 48)

== system MX_Gateway/MX_Gtwy_Control/Inputs/EOP/SensorInput ==
  adin_EOP : Inport  OutDataTypeStr=uint16
  ain_SensorSupply1 : Inport  Port=2
  ain_SensorSupply2 : Inport  Port=3
  state_SensorSupply1 : Inport  Port=4  OutDataTypeStr=uint8
  state_SensorSupply2 : Inport  Port=5  OutDataTypeStr=uint8
  state_Engine : Inport  Port=6  OutDataTypeStr=uint8
  flt_EngineSpeed : Inport  Port=7
  state_System : Inport  Port=8
  ecu_EOP : Inport  Port=9  OutDataTypeStr=uint8
  state_CommECU : Inport  Port=10  OutDataTypeStr=uint8
  Enable : EnablePort  PropagateVarSize=During execution
      settings: AttributesFormatString=<states=%<StatesWhenEnabling>>
  Compare To Constant : simulink/Logic and Bit Operations/Compare To Constant  relop===  const=NON_FUNCTIONAL  OutDataTypeStr=boolean  ZeroCross=off
  ECU Function : Constant  Value=CD_ECU_FUNCTION  OutDataTypeStr=uint8
  ErrorDetection : Stateflow chart  TreatAsAtomicUnit=on
  Sensor_Calculations : Subsystem  TreatAsAtomicUnit=on
  percent : Lookup  InputValues=[0:150]  Table=MXGTW_EOPGAUGE  LookUpMeth=Use Input Nearest  OutDataTypeStr=uint16  RndMeth=Zero  SaturateOnIntegerOverflow=on
      settings: AttributesFormatString=input = %<InputValues>\noutput = %<OutputValues>\nlookup = %<LookUpMeth>\nrounding = %<RndMeth>
  state_EOPSr : Outport  InitialOutput=[INIT_NORMAL]
  flt_EOP : Outport  Port=2  InitialOutput=[0]
  gau_EOP : Outport  Port=3  InitialOutput=[0]
  rm_EOP : Outport  Port=4  InitialOutput=[0]
  level_WarningEOP : Outport  Port=5  InitialOutput=[0]
  level_ShutdownEOP : Outport  Port=6  InitialOutput=[0]
  wires:
  state_System/1 -> ErrorDetection/7(state_System)  "state_System"
  percent/1 -> gau_EOP/1  "gau_EOP"
  state_Engine/1 -> ErrorDetection/4(state_Engine)  "state_Engine"
  Sensor_Calculations/3(state_SensorSupply) -> ErrorDetection/3(state_SensorSupply)  "state_SensorSupply"
  ErrorDetection/1(state_EOPSr) -> state_EOPSr/1  "state_EOPSr"
  ErrorDetection/2(flt_EOP) -> flt_EOP/1, percent/1  "flt_EOP"
  ecu_EOP/1 -> ErrorDetection/8(ecu_EOP)  "ecu_EOP"
  state_CommECU/1 -> ErrorDetection/9(state_CommECU)  "state_CommECU"
  adin_EOP/1 -> Sensor_Calculations/1(adin_EOP)  "adin_EOP"
  ain_SensorSupply1/1 -> Sensor_Calculations/2(ain_SenosrSupply1)  "ain_SensorSupply1"
  ain_SensorSupply2/1 -> Sensor_Calculations/3(ain_SensorSupply2)  "ain_SensorSupply2"
  state_SensorSupply1/1 -> Sensor_Calculations/4(state_SensorSupply1)  "state_SensorSupply1"
  state_SensorSupply2/1 -> Sensor_Calculations/5(state_SensorSupply2)  "state_SensorSupply2"
  flt_EngineSpeed/1 -> Sensor_Calculations/6(flt_EngineSpeed)  "flt_EngineSpeed"
  Sensor_Calculations/1(rm_EOP) -> ErrorDetection/1(rm_EOP), rm_EOP/1  "rm_EOP"
  Sensor_Calculations/2(flt_EOP_in) -> ErrorDetection/2(flt_EOP_in)  "flt_EOP_in"
  Sensor_Calculations/4(level_WarningEOP) -> ErrorDetection/5(level_WarningEOP), level_WarningEOP/1  "level_WarningEOP"
  Sensor_Calculations/5(level_ShutdownEOP) -> ErrorDetection/6(level_ShutdownEOP), level_ShutdownEOP/1  "level_ShutdownEOP"
  ECU Function/1 -> Compare To Constant/1
  Compare To Constant/1 -> Sensor_Calculations/Enable

== system MX_Gateway/MX_Gtwy_Control/Inputs/EOP/SwitchInput ==
  adin_EOP : Inport
  state_Engine : Inport  Port=2
  Enable : EnablePort  PropagateVarSize=During execution
      settings: AttributesFormatString=<states=%<StatesWhenEnabling>>
  Constant : Constant  Value=CD_EOP_SWITCH_GAUGE_VALUE
  Constant1 : Constant  Value=CD_EOP_SWITCH_PRESSURE_VALUE
  SwitchInput : Stateflow chart  TreatAsAtomicUnit=on
  state_EOPSw : Outport  InitialOutput=[INIT_NORMAL]
  flt_EOP : Outport  Port=2  InitialOutput=[0]
  gau_EOP : Outport  Port=3  InitialOutput=[0]
  wires:
  SwitchInput/1(state_EOPSw) -> state_EOPSw/1  "state_EOPSw"
  adin_EOP/1 -> SwitchInput/1(adin_EOP)  "adin_EOP"
  state_Engine/1 -> SwitchInput/2(state_Engine)  "state_Engine"
  Constant/1 -> gau_EOP/1
  Constant1/1 -> flt_EOP/1

== system MX_Gateway/MX_Gtwy_Control/Inputs/EngineSpeed/calculate_engine_speed ==
  freq_EngineSpeed : Inport
  Enable : EnablePort  StatesWhenEnabling=reset
  Divide : Product  Inputs=*/  OutDataTypeStr=uint16
  Flywheel_Teeth : Constant  Value=CD_FLYWHEEL_TEETH  OutDataTypeStr=uint16
  Gain1 : Gain  Gain=60  ParamDataTypeStr=uint8  OutDataTypeStr=uint32
  Period_Counts_Per_Second : Constant  Value=PERIOD_COUNTS_PER_SECOND  OutDataTypeStr=uint32
  Product : Product  OutDataTypeStr=uint32  RndMeth=Zero
  calc_EngineSpeed : Outport  InitialOutput=[0]
  wires:
  Gain1/1 -> Divide/1
  Period_Counts_Per_Second/1 -> Gain1/1
  Divide/1 -> calc_EngineSpeed/1  "calc_EngineSpeed"
  Product/1 -> Divide/2
  Flywheel_Teeth/1 -> Product/2  "FlywheelTeeth"
  freq_EngineSpeed/1 -> Product/1  "freq_EngineSped"

== system MX_Gateway/MX_Gtwy_Control/Inputs/HydOilTemp/SenderState ==
  adin_HOT : Inport  OutDataTypeStr=uint16
  ain_SensorSupply1 : Inport  Port=2  OutDataTypeStr=uint16
  state_SensorSupply1 : Inport  Port=3  OutDataTypeStr=uint8
  state_System : Inport  Port=4
  state_Engine : Inport  Port=5
  Enable : EnablePort  PropagateVarSize=During execution
      settings: AttributesFormatString=<state=%<StatesWhenEnabling>>
  Compare To state_System : simulink/Logic and Bit Operations/Compare To Constant  relop===  const=SYS_NORMAL  OutDataTypeStr=boolean  ZeroCross=off
  Data Type Conversion1 : DataTypeConversion  OutDataTypeStr=uint16
  ErrorDetection : Stateflow chart  TreatAsAtomicUnit=on
  GaugeTable : Lookup  InputValues=[0:340]  Table=MXGTW_HOTGAUGE  LookUpMeth=Use Input Nearest  OutDataTypeStr=uint16  RndMeth=Zero  SaturateOnIntegerOverflow=on
      settings: AttributesFormatString=input = %<InputValues>\noutput = %<OutputValues>\nlookup = %<LookUpMeth>\nrounding = %<RndMeth>
  HOT_Calculations : Subsystem
  RealWorldTable : Lookup  InputValues=MXGTW_HOTSND  Table=[0:340]  LookUpMeth=Use Input Nearest  OutDataTypeStr=uint16  RndMeth=Zero  SaturateOnIntegerOverflow=on
      settings: AttributesFormatString=input = %<InputValues>\noutput = %<OutputValues>\nlookup = %<LookUpMeth>\nrounding = %<RndMeth>
  state_HOT : Outport  InitialOutput=[INIT_NORMAL]
  flt_HOT : Outport  Port=2  InitialOutput=[0]
  gau_HOT : Outport  Port=3  InitialOutput=[0]
  rm_HOT : Outport  Port=4  InitialOutput=[0]
  wires:
  state_SensorSupply1/1 -> ErrorDetection/3(state_SensorSupply1)  "state_SensorSupply1"
  ErrorDetection/1(state_HOT) -> state_HOT/1  "state_HOT"
  GaugeTable/1 -> gau_HOT/1  "gau_HOT"
  RealWorldTable/1 -> ErrorDetection/2(flt_HOT_in)  "flt_HOT_in"
  ain_SensorSupply1/1 -> HOT_Calculations/2(ain_SensorSupply1)  "ain_SensorSupply1"
  adin_HOT/1 -> HOT_Calculations/1(adin_HOT)  "adin_HOT"
  HOT_Calculations/2(rmFlt_HOT) -> Data Type Conversion1/1
  [state_System] -> ErrorDetection/4(state_System)  "state_System"
  state_Engine/1 -> ErrorDetection/5(state_Engine)  "state_Engine"
  ErrorDetection/2(flt_HOT) -> flt_HOT/1, GaugeTable/1  "flt_HOT"
  state_System/1 -> [state_System]  "state_System"
  HOT_Calculations/1(rm_HOT) -> ErrorDetection/1(rm_HOT), rm_HOT/1  "rm_HOT"
  [state_System] -> HOT_Calculations/3(state_System)  "state_System"
  Data Type Conversion1/1 -> RealWorldTable/1
  Compare To state_System/1 -> HOT_Calculations/Enable
  [state_System] -> Compare To state_System/1  "state_System"

== system MX_Gateway/MX_Gtwy_Control/Inputs/HydOilTemp/SwitchState ==
  adin_HOT : Inport
  state_System : Inport  Port=2
  Enable : EnablePort  PropagateVarSize=During execution
      settings: AttributesFormatString=<state=%<StatesWhenEnabling>>
  State_Switch : Stateflow chart  TreatAsAtomicUnit=on
  state_HOT : Outport  InitialOutput=[INIT_NORMAL]
  wires:
  State_Switch/1(state_HOT) -> state_HOT/1  "state_HOT"
  adin_HOT/1 -> State_Switch/1(adin_HOT)  "adin_HOT"
  state_System/1 -> State_Switch/2(state_System)  "state_System"

== system MX_Gateway/MX_Gtwy_Control/Inputs/Joystick_Pots/Left ==
  Pot_Raw : Inport
  J1939_Joystick_Pot : Subsystem
  Pot_Cmd : Outport
  wires:
  Pot_Raw/1 -> J1939_Joystick_Pot/1(In1)
  J1939_Joystick_Pot/1(Out1) -> Pot_Cmd/1

== system MX_Gateway/MX_Gtwy_Control/Inputs/Joystick_Pots/Right ==
  Pot_Raw : Inport
  Buttons_5_8 : Inport  Port=2
  Buttons : Subsystem
  J1939_Joystick_Pot : Subsystem
  Pot_Cmd : Outport
  Trigger : Outport  Port=2
  wires:
  Pot_Raw/1 -> J1939_Joystick_Pot/1(In1)
  J1939_Joystick_Pot/1(Out1) -> Pot_Cmd/1
  Buttons_5_8/1 -> Buttons/1(Buttons_5_8)
  Buttons/1(Trigger) -> Trigger/1

== system MX_Gateway/MX_Gtwy_Control/Inputs/Load_Moment/Load_Moment_Sensor ==
  ain_LoadMoment : Inport
  ain_SensorSupply2 : Inport  Port=2
  state_SensorSupply2 : Inport  Port=3
  din_OWDEnableSw : Inport  Port=4
  Enable : EnablePort  StatesWhenEnabling=reset  PropagateVarSize=During execution
  Load_Moment : Stateflow chart  TreatAsAtomicUnit=on
  Pressure_Transform : Subsystem
  state_LoadMoment : Outport  OutputWhenDisabled=reset  InitialOutput=[INIT_NORMAL]
  bar_LoadMoment : Outport  Port=2  OutputWhenDisabled=reset  InitialOutput=[0]
  volts_LoadMoment : Outport  Port=3  OutputWhenDisabled=reset  InitialOutput=[0]
  wires:
  ain_LoadMoment/1 -> Pressure_Transform/1(ain_LoadMoment)
  ain_SensorSupply2/1 -> Pressure_Transform/2(ain_SensorSupply2)
  Load_Moment/1(state_LoadMoment) -> state_LoadMoment/1
  Pressure_Transform/1(bar_LoadMoment) -> Load_Moment/1(bar_LoadMoment), bar_LoadMoment/1
  Pressure_Transform/2(volts_LoadMoment) -> Load_Moment/2(volts_LoadMoment), volts_LoadMoment/1
  state_SensorSupply2/1 -> Load_Moment/3(state_SensorSupply)
  din_OWDEnableSw/1 -> Load_Moment/4(din_OWDEnableSw)

== system MX_Gateway/MX_Gtwy_Control/Inputs/Load_Moment/Load_Moment_Switch ==
  ain_LoadMoment : Inport
  Enable : EnablePort  StatesWhenEnabling=reset  PropagateVarSize=During execution
  Load_Moment : Stateflow chart  TreatAsAtomicUnit=on
  state_LoadMoment : Outport  OutputWhenDisabled=reset  InitialOutput=[INIT_NORMAL]
  wires:
  ain_LoadMoment/1 -> Load_Moment/1(ain_LoadMoment)
  Load_Moment/1(state_LoadMoment) -> state_LoadMoment/1

== system MX_Gateway/MX_Gtwy_Control/Inputs/Load_Sense/Load_Sense ==
  ain_SensorSupply2 : Inport
  ain_LoadSense : Inport  Port=2
  ain_SensorSupply1 : Inport  Port=3
  state_SensorSupply1 : Inport  Port=4
  state_SensorSupply2 : Inport  Port=5
  Enable : EnablePort  StatesWhenEnabling=reset
  Pressure_Monitor : Stateflow chart  RequirementInfo={} %GIDa_fcd67128_45de_4c87_8df5_fe08704550a5  TreatAsAtomicUnit=on
  Pressure_Transform : Subsystem
  psi_LoadSense : Outport  OutputWhenDisabled=reset  InitialOutput=[0]
  bar_LoadSense : Outport  Port=2  OutputWhenDisabled=reset  InitialOutput=[0]
  state_LoadSense : Outport  Port=3  OutputWhenDisabled=reset  InitialOutput=[8]
  volts_LoadSense : Outport  Port=4  OutputWhenDisabled=reset  InitialOutput=[0]
  wires:
  Pressure_Transform/1(psi_LoadSense) -> psi_LoadSense/1
  Pressure_Monitor/1(state_LoadSense) -> state_LoadSense/1
  ain_LoadSense/1 -> Pressure_Transform/1(ain_LoadSense)
  ain_SensorSupply2/1 -> Pressure_Transform/2(ain_SensorSupply2)
  ain_SensorSupply1/1 -> Pressure_Transform/3(ain_SensorSupply1)
  Pressure_Transform/2(bar_LoadSense) -> bar_LoadSense/1
  Pressure_Transform/3(volts_LoadSense) -> volts_LoadSense/1, Pressure_Monitor/1(volts_LoadSense)
  Pressure_Transform/4(state_SensorSupply) -> Pressure_Monitor/2(state_SensorSupply)
  state_SensorSupply1/1 -> Pressure_Transform/4(state_SensorSupply1)
  state_SensorSupply2/1 -> Pressure_Transform/5(state_SensorSupply2)

== system MX_Gateway/MX_Gtwy_Control/Inputs/Offset_Pot/CAN_Pot ==
  state_Console : Inport
  state_Engine : Inport  Port=2
  state_JoystickEnable : Inport  Port=3
  msgAge_BJM2Left : Inport  Port=4
  cmd_PotLeft : Inport  Port=5
  Enable : EnablePort  StatesWhenEnabling=reset  PropagateVarSize=During execution
  Constant : Constant  Value=500  OutDataTypeStr=int16
  Data Type Conversion1 : DataTypeConversion  OutDataTypeStr=uint16
  Gain : Gain  Gain=1/2  OutDataTypeStr=int16
  Pot : Stateflow chart  TreatAsAtomicUnit=on
  Sum : Sum  Inputs=|++
  Unary Minus : UnaryMinus
  state_OffsetPot : Outport  OutputWhenDisabled=reset  InitialOutput=[INIT_NORMAL]
  position_OffsetPot : Outport  Port=2  OutputWhenDisabled=reset  InitialOutput=[500]
  rm_OffsetPot : Outport  Port=3  OutputWhenDisabled=reset  InitialOutput=[500]
  wires:
  Pot/1(state_OffsetPot) -> state_OffsetPot/1
  state_Console/1 -> Pot/3(state_Console)
  Pot/2(position_OffsetPotOut) -> position_OffsetPot/1
  state_Engine/1 -> Pot/4(state_Engine)
  state_JoystickEnable/1 -> Pot/5(state_JoystickEnable)
  msgAge_BJM2Left/1 -> Pot/2(msgAge_BJM2Left)
  cmd_PotLeft/1 -> Unary Minus/1
  Data Type Conversion1/1 -> rm_OffsetPot/1
  Constant/1 -> Sum/2
  Sum/1 -> Data Type Conversion1/1, Pot/1(rm_OffsetPot)
  Gain/1 -> Sum/1
  Unary Minus/1 -> Gain/1

== system MX_Gateway/MX_Gtwy_Control/Inputs/Offset_Pot/Normal_Pot ==
  ain_OffsetPot : Inport
  ain_SensorSupply2 : Inport  Port=2
  state_Console : Inport  Port=3
  state_Engine : Inport  Port=4
  state_JoystickEnable : Inport  Port=5
  state_SensorSupply2 : Inport  Port=6
  Enable : EnablePort  StatesWhenEnabling=reset  PropagateVarSize=During execution
  Data Type Conversion : DataTypeConversion  OutDataTypeStr=int16
  Pot : Stateflow chart  TreatAsAtomicUnit=on
  Pot Ratio : Subsystem
  state_OffsetPot : Outport  OutputWhenDisabled=reset  InitialOutput=[INIT_NORMAL]
  position_OffsetPot : Outport  Port=2  OutputWhenDisabled=reset  InitialOutput=[500]
  rm_OffsetPot : Outport  Port=3  OutputWhenDisabled=reset  InitialOutput=[500]
  wires:
  ain_OffsetPot/1 -> Pot Ratio/1(ain_OffsetPot)
  Pot/1(state_OffsetPot) -> state_OffsetPot/1
  ain_SensorSupply2/1 -> Pot Ratio/2(ain_SensorSupply2)
  state_Console/1 -> Pot/3(state_Console)
  Pot/2(position_OffsetPotOut) -> position_OffsetPot/1
  state_Engine/1 -> Pot/4(state_Engine)
  Data Type Conversion/1 -> Pot/1(rm_OffsetPot)
  Pot Ratio/1(rm_OffsetPot) -> rm_OffsetPot/1, Data Type Conversion/1
  state_JoystickEnable/1 -> Pot/5(state_JoystickEnable)
  state_SensorSupply2/1 -> Pot/2(state_SensorSupply)

== system MX_Gateway/MX_Gtwy_Control/Inputs/Throttle/FastTravelThrottleLimit ==
  in_Throttle : Inport
  adin_TravelPressureSwitch : Inport  Port=2
  Throttle_Limiter : Stateflow chart  TreatAsAtomicUnit=on
  out_Throttle : Outport
  wires:
  in_Throttle/1 -> Throttle_Limiter/1(in_Throttle)
  adin_TravelPressureSwitch/1 -> Throttle_Limiter/2(adin_TravelPressureSwitch)
  Throttle_Limiter/1(out_Throttle) -> out_Throttle/1

== system MX_Gateway/MX_Gtwy_Control/Inputs/Throttle/Primary Throttle ==
  note: Medium and Large Frame have throttle extended at low idle
      | Small Frame has throttle retracted at low idle
  ain_Throttle : Inport
  ain_SensorSupply2 : Inport  Port=2
  state_EcoMode : Inport  Port=3
  CompareToConstant1 : simulink/Logic and Bit Operations/Compare To Constant  relop===  const=NON_FUNCTIONAL  OutDataTypeStr=boolean  ZeroCross=off
  Data Type Conversion1 : DataTypeConversion  OutDataTypeStr=uint16
  Data Type Conversion2 : DataTypeConversion
  Data Type Conversion4 : DataTypeConversion
  Data Type Conversion5 : DataTypeConversion
  FILTER_GAIN : Constant  Value=CD_THROTTLE_PRIMARY_FILTER_GAIN  OutDataTypeStr=uint8  SampleTime=-1
  LP_Filter_Adj_Gain : MX_Library/LP_Filter_Adj_Gain
  LP_Filter_Adj_Gain1 : MX_Library/LP_Filter_Adj_Gain
  Lookup Table Dynamic : simulink/Lookup Tables/Lookup Table Dynamic  LookUpMeth=Interpolation-Use End Values  OutDataType=fixdt(0,16,0)  OutScaling=[]  OutputDataTypeScalingMode=Specify via dialog  OutDataTypeStr=fixdt(0,16,0)  DoSatur=off
      settings: SourceProductName=Simulink
  Mux : Mux  Inputs=2
  Mux1 : Mux  Inputs=2
  Ratiometric_Converter : MX_Library/Ratiometric_Converter  multiplier=CD_THROTTLE_PRIMARY_RATIO_MULTIPLIER
  Remove_OOR_Levels : MX_Library/Remove_OOR_Levels  OORL_LEVEL=50  OORH_LEVEL=950
  Sum : Sum  Inputs=|+-
  Switch : Switch  Criteria=u2 ~= 0
  Switch1 : Switch  Criteria=u2 ~= 0
  Switch2 : Switch  Criteria=u2 ~= 0
  THROTTLE_OUTPUT_HIGH : Constant  Value=CD_THROTTLE_PRI_OUTPUT_HIGH  OutDataTypeStr=uint16  SampleTime=-1
  THROTTLE_OUTPUT_HIGH1 : Constant  Value=CD_ECO_MODE_THROTTLE_REDUCTION  OutDataTypeStr=uint16  SampleTime=-1
  THROTTLE_OUTPUT_LOW : Constant  Value=CD_THROTTLE_PRI_OUTPUT_LOW  OutDataTypeStr=uint16  SampleTime=-1
  THROTTLE_RATIO_HIGH : Constant  Value=CD_THROTTLE_PRI_RATIO_HIGH  OutDataTypeStr=uint16  SampleTime=-1
  THROTTLE_RATIO_LOW : Constant  Value=CD_THROTTLE_PRI_RATIO_LOW  OutDataTypeStr=uint16  SampleTime=-1
  THROTTLE_RETRACTED1 : Constant  Value=CD_CAN_THROTTLE_REVERSE  OutDataTypeStr=uint8  SampleTime=srate100
  filter_level : Constant  Value=3  OutDataTypeStr=uint8  SampleTime=-1
  filter_level1 : Constant  Value=0  OutDataTypeStr=uint16  SampleTime=-1
  pos_Throttle : Outport
  rm_Throttle : Outport  Port=2
  wires:
  Data Type Conversion5/1 -> LP_Filter_Adj_Gain1/2
  filter_level/1 -> LP_Filter_Adj_Gain1/1
  LP_Filter_Adj_Gain1/1 -> Data Type Conversion4/1
  Data Type Conversion2/1 -> LP_Filter_Adj_Gain/2  "ratiometric"
  Remove_OOR_Levels/1 -> Data Type Conversion2/1
  Data Type Conversion4/1 -> rm_Throttle/1  "rm_Throttle"
  Ratiometric_Converter/1 -> Data Type Conversion5/1, Remove_OOR_Levels/1  "ratiometric"
  FILTER_GAIN/1 -> LP_Filter_Adj_Gain/1  "Gain"
  Data Type Conversion1/1 -> Lookup Table Dynamic/1  "fltRatio"
  LP_Filter_Adj_Gain/1 -> Data Type Conversion1/1  "fltRatio"
  ain_SensorSupply2/1 -> Ratiometric_Converter/2  "ain_SensorSupply2"
  ain_Throttle/1 -> Ratiometric_Converter/1
  THROTTLE_RATIO_LOW/1 -> Mux/1
  Mux/1 -> Lookup Table Dynamic/2  "data_Ratio"
  Mux1/1 -> Lookup Table Dynamic/3  "data_Output"
  THROTTLE_RATIO_HIGH/1 -> Mux/2
  THROTTLE_OUTPUT_HIGH/1 -> Sum/1
  Switch/1 -> Sum/2
  THROTTLE_OUTPUT_HIGH1/1 -> Switch/1
  filter_level1/1 -> Switch/3
  state_EcoMode/1 -> Switch/2
  THROTTLE_RETRACTED1/1 -> CompareToConstant1/1
  Switch1/1 -> Mux1/1
  Switch2/1 -> Mux1/2
  THROTTLE_OUTPUT_LOW/1 -> Switch1/1, Switch2/3
  Sum/1 -> Switch2/1, Switch1/3
  CompareToConstant1/1 -> Switch1/2, Switch2/2
  Lookup Table Dynamic/1 -> pos_Throttle/1  "pos_Throttle"

== system MX_Gateway/MX_Gtwy_Control/Inputs/VBattery/LP_Filter_Adj_Gain ==
  Gain : Inport
  Input : Inport  Port=2
  LP_Fitler_Adj_Gain : Stateflow chart  TreatAsAtomicUnit=on
  Output : Outport
  wires:
  LP_Fitler_Adj_Gain/1(Output) -> Output/1
  Input/1 -> LP_Fitler_Adj_Gain/2(Input)
  Gain/1 -> LP_Fitler_Adj_Gain/1(Gain)

== system MX_Gateway/MX_Gtwy_Control/Inputs/VBattery/LP_Filter_Adj_Gain1 ==
  Gain : Inport
  Input : Inport  Port=2
  LP_Fitler_Adj_Gain : Stateflow chart  TreatAsAtomicUnit=on
  Output : Outport
  wires:
  LP_Fitler_Adj_Gain/1(Output) -> Output/1
  Input/1 -> LP_Fitler_Adj_Gain/2(Input)
  Gain/1 -> LP_Fitler_Adj_Gain/1(Gain)
